simulink model "Lab2_simulink_plc"  (R2023b)
settings: Solver=FixedStepAuto  StartTime=0.0  StopTime=inf  RelTol=1e-3
InitFcn:
  | d13_init.PRE = 50;
undefined symbols (not defined in the file or in its folder): BOOL, DINT, OTL, slplc
libraries: arduinolib x9
(part 3 of 31)

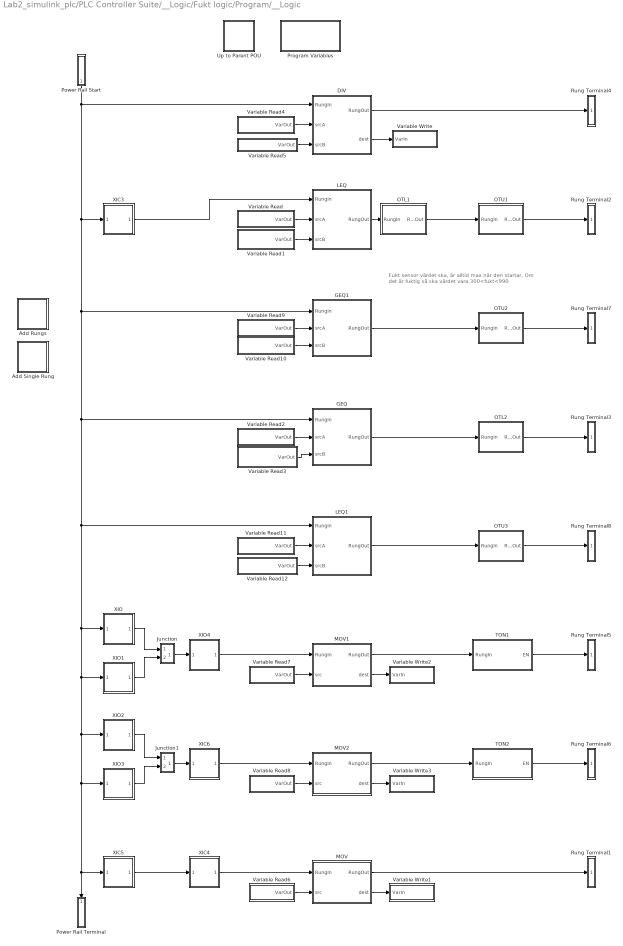
== system Lab2_simulink_plc/PLC Controller Suite/__Logic/Fukt logic/Program/__Logic ==
  note: Fukt sensor värdet ska, är alltid max när den startar,
      | Om det är fuktig så ska värdet vara 300<fukt<990
  Add Rungs : Subsystem  DisableCoverage=on  TreatAsAtomicUnit=on  mask{PLCRungNumberToAdd=2, PLCBlockType=AddRungs}
      mask prompts: PLCRungNumberToAdd "Rung Number:"
      mask Help: web([docroot '/plccoder/ref/addrungs' '.html']);
      mask DialogControl PLCAddRungs Prompt: Add Rungs
      mask DialogControl PLCRungAddButton Prompt: Add
      mask DialogControl PLCRungAddButton Callback: slplc.callbacks.addLadderRungs(gcb);
      mask Display: disp(sprintf(['Add' newline 'Rungs']));
  Add Single Rung : Subsystem  DisableCoverage=on  TreatAsAtomicUnit=on  mask{PLCRungNumberToAdd=1, PLCBlockType=AddSingleRung}
      settings: OpenFcn=slplc.callbacks.addLadderRungs(gcb);
      mask Help: web([docroot '/plccoder/ref/addsinglerung' '.html']);
      mask DialogControl PLCAddRung Prompt: Add Single Rung
      mask Display: disp(sprintf(['Add' newline '1 Rung']));
  DIV : Subsystem  Priority=4  TreatAsAtomicUnit=on  mask{PLCBlockType=DIV, PLCPOUType=stdFC}
      mask Help: web([docroot '/plccoder/ref/div' '.html']);
      mask DialogControl PLCBlockTitle Prompt: Divide (DIV)
      mask DialogControl PLCBlockDesc Prompt: Divide Source A by Source B.
      mask Display: disp('DIV');
  GEQ : Subsystem  Priority=21  TreatAsAtomicUnit=on  mask{PLCBlockType=GEQ, PLCPOUType=stdFC}
      mask Help: web([docroot '/plccoder/ref/geq' '.html']);
      mask DialogControl PLCBlockTitle Prompt: Greater Than or Equal To (GEQ)
      mask DialogControl PLCBlockDesc Prompt: Test whether Source A is greater than or equal to Source B.
      mask Display: disp('GEQ');
  GEQ1 : Subsystem  Priority=16  TreatAsAtomicUnit=on  mask{PLCBlockType=GEQ, PLCPOUType=stdFC}
      mask Help: web([docroot '/plccoder/ref/geq' '.html']);
      mask DialogControl PLCBlockTitle Prompt: Greater Than or Equal To (GEQ)
      mask DialogControl PLCBlockDesc Prompt: Test whether Source A is greater than or equal to Source B.
      mask Display: disp('GEQ');
  Junction : Subsystem  Priority=31  DisableCoverage=on  TreatAsAtomicUnit=on  mask{PLCBlockType=Junction, PLCPOUType=BranchJunction}
      mask Help: web([docroot '/plccoder/ref/junction' '.html']);
      mask DialogControl PLCBlockTitle Prompt: Rung Merge Point
      mask DialogControl PLCBlockDesc Prompt: This is the ladder diagram rung merge point.
  Junction1 : Subsystem  Priority=40  DisableCoverage=on  TreatAsAtomicUnit=on  mask{PLCBlockType=Junction, PLCPOUType=BranchJunction}
      mask Help: web([docroot '/plccoder/ref/junction' '.html']);
      mask DialogControl PLCBlockTitle Prompt: Rung Merge Point
      mask DialogControl PLCBlockDesc Prompt: This is the ladder diagram rung merge point.
  LEQ : Subsystem  Priority=10  TreatAsAtomicUnit=on  mask{PLCBlockType=LEQ, PLCPOUType=stdFC}
      mask Help: web([docroot '/plccoder/ref/leq' '.html']);
      mask DialogControl PLCBlockTitle Prompt: Less Than or Equal To (LEQ)
      mask DialogControl PLCBlockDesc Prompt: Test whether Source A is less than or equal to Source B.
      mask Display: disp('LEQ');
  LEQ1 : Subsystem  Priority=26  TreatAsAtomicUnit=on  mask{PLCBlockType=LEQ, PLCPOUType=stdFC}
      mask Help: web([docroot '/plccoder/ref/leq' '.html']);
      mask DialogControl PLCBlockTitle Prompt: Less Than or Equal To (LEQ)
      mask DialogControl PLCBlockDesc Prompt: Test whether Source A is less than or equal to Source B.
      mask Display: disp('LEQ');
  MOV : Subsystem  Priority=50  TreatAsAtomicUnit=on  mask{PLCBlockType=MOV, PLCPOUType=stdFC}
      mask Help: web([docroot '/plccoder/ref/mov' '.html']);
      mask DialogControl PLCBlockTitle Prompt: Move (MOV)
      mask DialogControl PLCBlockDesc Prompt: Move a copy of the Source to the Destination. The Source remains unchanged.
      mask Display: disp('MOV');
  MOV1 : Subsystem  Priority=34  TreatAsAtomicUnit=on  mask{PLCBlockType=MOV, PLCPOUType=stdFC}
      mask Help: web([docroot '/plccoder/ref/mov' '.html']);
      mask DialogControl PLCBlockTitle Prompt: Move (MOV)
      mask DialogControl PLCBlockDesc Prompt: Move a copy of the Source to the Destination. The Source remains unchanged.
      mask Display: disp('MOV');
  MOV2 : Subsystem  Priority=43  TreatAsAtomicUnit=on  mask{PLCBlockType=MOV, PLCPOUType=stdFC}
      mask Help: web([docroot '/plccoder/ref/mov' '.html']);
      mask DialogControl PLCBlockTitle Prompt: Move (MOV)
      mask DialogControl PLCBlockDesc Prompt: Move a copy of the Source to the Destination. The Source remains unchanged.
      mask Display: disp('MOV');
  OTL1 : Subsystem  Priority=11  TreatAsAtomicUnit=on  mask{PLCOperandTag=fukt_low, PLCBlockType=OTL, PLCPOUType=Coil, PLCOperandExpression=xxx_PLC_VAR_fukt_low}
      mask prompts: PLCOperandTag "Operand Tag:"
      mask init:
      | slplc.callbacks.initInstruction(gcb);
      settings: AttributesFormatString=%<PLCOperandTag>
      mask Help: web([docroot '/plccoder/ref/otl' '.html']);
      mask DialogControl PLCBlockTitle Prompt: Output Latch (OTL)
      mask Display: disp('L');
  OTL2 : Subsystem  Priority=22  TreatAsAtomicUnit=on  mask{PLCOperandTag=fukt_high, PLCBlockType=OTL, PLCPOUType=Coil, PLCOperandExpression=xxx_PLC_VAR_fukt_high}
      mask prompts: PLCOperandTag "Operand Tag:"
      mask init:
      | slplc.callbacks.initInstruction(gcb);
      settings: AttributesFormatString=%<PLCOperandTag>
      mask Help: web([docroot '/plccoder/ref/otl' '.html']);
      mask DialogControl PLCBlockTitle Prompt: Output Latch (OTL)
      mask Display: disp('L');
  OTU1 : Subsystem  Priority=12  TreatAsAtomicUnit=on  mask{PLCOperandTag=fukt_polling, PLCBlockType=OTU, PLCPOUType=Coil, PLCOperandExpression=xxx_PLC_VAR_fukt_polling}
      mask prompts: PLCOperandTag "Operand Tag:"
      mask init:
      | slplc.callbacks.initInstruction(gcb);
      settings: AttributesFormatString=%<PLCOperandTag>
      mask Help: web([docroot '/plccoder/ref/otu' '.html']);
      mask DialogControl PLCBlockTitle Prompt: Output Unlatch (OTU)
      mask Display: disp('U');
  OTU2 : Subsystem  Priority=17  TreatAsAtomicUnit=on  mask{PLCOperandTag=fukt_low, PLCBlockType=OTU, PLCPOUType=Coil, PLCOperandExpression=xxx_PLC_VAR_fukt_low}
      mask prompts: PLCOperandTag "Operand Tag:"
      mask init:
      | slplc.callbacks.initInstruction(gcb);
      settings: AttributesFormatString=%<PLCOperandTag>
      mask Help: web([docroot '/plccoder/ref/otu' '.html']);
      mask DialogControl PLCBlockTitle Prompt: Output Unlatch (OTU)
      mask Display: disp('U');
  OTU3 : Subsystem  Priority=27  TreatAsAtomicUnit=on  mask{PLCOperandTag=fukt_high, PLCBlockType=OTU, PLCPOUType=Coil, PLCOperandExpression=xxx_PLC_VAR_fukt_high}
      mask prompts: PLCOperandTag "Operand Tag:"
      mask init:
      | slplc.callbacks.initInstruction(gcb);
      settings: AttributesFormatString=%<PLCOperandTag>
      mask Help: web([docroot '/plccoder/ref/otu' '.html']);
      mask DialogControl PLCBlockTitle Prompt: Output Unlatch (OTU)
      mask Display: disp('U');
  Power Rail Start : Subsystem  Priority=1  DisableCoverage=on  TreatAsAtomicUnit=on  mask{PLCBlockType=PowerRailStart}
      mask Help: web([docroot '/plccoder/ref/powerrailstart' '.html']);
      mask DialogControl PLCBlockTitle Prompt: Power Rail Start
      mask DialogControl PLCBlockDesc Prompt: This is the ladder diagram left power rail start block.
  Power Rail Terminal : Subsystem  DisableCoverage=on  TreatAsAtomicUnit=on  mask{PLCBlockType=PowerRailTerminal}
      mask Help: web([docroot '/plccoder/ref/powerrailterminal' '.html']);
      mask DialogControl PLCBlockTitle Prompt: Power Rail Terminal
      mask DialogControl PLCBlockDesc Prompt: This is the ladder diagram left power rail terminal block.
  Program Variables : Subsystem  Tag=ProgramVariableSS  DisableCoverage=on  TreatAsAtomicUnit=on  mask{PLCVarFukt_constant_highName=Fukt_constant_high, PLCVarFukt_constant_highScope=Local, PLCVarFukt_constant_highPortType=Hidden, PLCVarFukt_constant_highPortIndex=12, PLCVarFukt_constant_highDataType=DINT, PLCVarFukt_constant_highSize=1, PLCVarFukt_constant_highInitialValue=900, PLCVarFukt_constant_highToDelete=off, PLCVarFukt_constant_lowName=Fukt_constant_low, PLCVarFukt_constant_lowScope=Local, PLCVarFukt_constant_lowPortType=Hidden, PLCVarFukt_constant_lowPortIndex=12, PLCVarFukt_constant_lowDataType=DINT, PLCVarFukt_constant_lowSize=1, PLCVarFukt_constant_lowInitialValue=300, PLCVarFukt_constant_lowToDelete=off, PLCVarconstantName=constant, PLCVarconstantScope=Local, PLCVarconstantPortType=Hidden, PLCVarconstantPortIndex=12, PLCVarconstantDataType=uint16, PLCVarconstantSize=1, PLCVarconstantInitialValue=4, PLCVarconstantToDelete=off, PLCVarduty_cycleName=duty_cycle, PLCVarduty_cycleScope=Local, PLCVarduty_cyclePortType=Hidden, PLCVarduty_cyclePortIndex=12, PLCVarduty_cycleDataType=uint8, PLCVarduty_cycleSize=1, PLCVarduty_cycleInitialValue=0, PLCVarduty_cycleToDelete=off, PLCVarfukt_highName=fukt_high, PLCVarfukt_highScope=Local, PLCVarfukt_highPortType=Hidden, PLCVarfukt_highPortIndex=12, PLCVarfukt_highDataType=BOOL, PLCVarfukt_highSize=1, PLCVarfukt_highInitialValue=false, PLCVarfukt_highToDelete=off, PLCVarfukt_lowName=fukt_low, PLCVarfukt_lowScope=Local, PLCVarfukt_lowPortType=Hidden, PLCVarfukt_lowPortIndex=12, PLCVarfukt_lowDataType=BOOL, PLCVarfukt_lowSize=1, PLCVarfukt_lowInitialValue=false, PLCVarfukt_lowToDelete=off, PLCVarorange_led_timer_offName=orange_led_timer_off, PLCVarorange_led_timer_offScope=Local, PLCVarorange_led_timer_offPortType=Hidden, PLCVarorange_led_timer_offPortIndex=12, PLCVarorange_led_timer_offDataType=Bus: TIMER, PLCVarorange_led_timer_offSize=1, PLCVarorange_led_timer_offInitialValue=orange_period, PLCVarorange_led_timer_offToDelete=off, PLCVarorange_led_timer_onName=orange_led_timer_on, PLCVarorange_led_timer_onScope=Local, PLCVarorange_led_timer_onPortType=Hidden, PLCVarorange_led_timer_onPortIndex=12, PLCVarorange_led_timer_onDataType=Bus: TIMER, PLCVarorange_led_timer_onSize=1, PLCVarorange_led_timer_onInitialValue=orange_period, PLCVarorange_led_timer_onToDelete=off, PLCVarorange_offName=orange_off, PLCVarorange_offScope=Local, PLCVarorange_offPortType=Hidden, PLCVarorange_offPortIndex=12, PLCVarorange_offDataType=uint8, PLCVarorange_offSize=1, PLCVarorange_offInitialValue=0, PLCVarorange_offToDelete=off, PLCVarFukt_value_copyName=Fukt_value_copy, PLCVarFukt_value_copyScope=External, PLCVarFukt_value_copyPortType=Hidden, PLCVarFukt_value_copyPortIndex=12, PLCVarFukt_value_copyDataType=DINT, PLCVarFukt_value_copySize=-1, PLCVarFukt_value_copyInitialValue=0, PLCVarFukt_value_copyToDelete=off, PLCVarOrangeLedName=OrangeLed, PLCVarOrangeLedScope=External, PLCVarOrangeLedPortType=Hidden, PLCVarOrangeLedPortIndex=12, PLCVarOrangeLedDataType=DINT, PLCVarOrangeLedSize=-1, PLCVarOrangeLedInitialValue=0, PLCVarOrangeLedToDelete=off, PLCVarfukt_pollingName=fukt_polling, PLCVarfukt_pollingScope=External, PLCVarfukt_pollingPortType=Hidden, PLCVarfukt_pollingPortIndex=12, PLCVarfukt_pollingDataType=DINT, PLCVarfukt_pollingSize=-1, PLCVarfukt_pollingInitialValue=0, PLCVarfukt_pollingToDelete=off}
      mask init:
      | slplc.callbacks.initPOUVarSS(gcb);
      settings: OpenFcn=slplc.callbacks.openProgramVariableSS(gcb);
      mask Help: web([docroot '/plccoder/ref/programvariables' '.html']);
      mask TypeOptions PLCVarFukt_constant_highScope: Local | External
      mask Callback PLCVarFukt_constant_highScope: slplc.callbacks.updatePOUVarSS(gcb);
      mask TypeOptions PLCVarFukt_constant_highPortType: Hidden | Inport | Outport
      mask Callback PLCVarFukt_constant_highPortType: slplc.callbacks.updatePOUVarSS(gcb);
      mask TypeOptions PLCVarFukt_constant_highPortIndex: 1 | 2 | 3 | 4 | 5 | 6 | 7 | 8 | 9 | 10 | 11 | 12
      mask TypeOptions PLCVarFukt_constant_lowScope: Local | External
      mask Callback PLCVarFukt_constant_lowScope: slplc.callbacks.updatePOUVarSS(gcb);
      mask TypeOptions PLCVarFukt_constant_lowPortType: Hidden | Inport | Outport
      mask Callback PLCVarFukt_constant_lowPortType: slplc.callbacks.updatePOUVarSS(gcb);
      mask TypeOptions PLCVarFukt_constant_lowPortIndex: 1 | 2 | 3 | 4 | 5 | 6 | 7 | 8 | 9 | 10 | 11 | 12
      mask TypeOptions PLCVarconstantScope: Local | External
      mask Callback PLCVarconstantScope: slplc.callbacks.updatePOUVarSS(gcb);
      mask TypeOptions PLCVarconstantPortType: Hidden | Inport | Outport
      mask Callback PLCVarconstantPortType: slplc.callbacks.updatePOUVarSS(gcb);
      mask TypeOptions PLCVarconstantPortIndex: 1 | 2 | 3 | 4 | 5 | 6 | 7 | 8 | 9 | 10 | 11 | 12
      mask TypeOptions PLCVarduty_cycleScope: Local | External
      mask Callback PLCVarduty_cycleScope: slplc.callbacks.updatePOUVarSS(gcb);
      mask TypeOptions PLCVarduty_cyclePortType: Hidden | Inport | Outport
      mask Callback PLCVarduty_cyclePortType: slplc.callbacks.updatePOUVarSS(gcb);
      mask TypeOptions PLCVarduty_cyclePortIndex: 1 | 2 | 3 | 4 | 5 | 6 | 7 | 8 | 9 | 10 | 11 | 12
      mask TypeOptions PLCVarfukt_highScope: Local | External
      mask Callback PLCVarfukt_highScope: slplc.callbacks.updatePOUVarSS(gcb);
      mask TypeOptions PLCVarfukt_highPortType: Hidden | Inport | Outport
      mask Callback PLCVarfukt_highPortType: slplc.callbacks.updatePOUVarSS(gcb);
      mask TypeOptions PLCVarfukt_highPortIndex: 1 | 2 | 3 | 4 | 5 | 6 | 7 | 8 | 9 | 10 | 11 | 12
      mask TypeOptions PLCVarfukt_lowScope: Local | External
      mask Callback PLCVarfukt_lowScope: slplc.callbacks.updatePOUVarSS(gcb);
      mask TypeOptions PLCVarfukt_lowPortType: Hidden | Inport | Outport
      mask Callback PLCVarfukt_lowPortType: slplc.callbacks.updatePOUVarSS(gcb);
      mask TypeOptions PLCVarfukt_lowPortIndex: 1 | 2 | 3 | 4 | 5 | 6 | 7 | 8 | 9 | 10 | 11 | 12
      mask TypeOptions PLCVarorange_led_timer_offScope: Local | External
      mask Callback PLCVarorange_led_timer_offScope: slplc.callbacks.updatePOUVarSS(gcb);
      mask TypeOptions PLCVarorange_led_timer_offPortType: Hidden | Inport | Outport
      mask Callback PLCVarorange_led_timer_offPortType: slplc.callbacks.updatePOUVarSS(gcb);
      mask TypeOptions PLCVarorange_led_timer_offPortIndex: 1 | 2 | 3 | 4 | 5 | 6 | 7 | 8 | 9 | 10 | 11 | 12
      mask TypeOptions PLCVarorange_led_timer_onScope: Local | External
      mask Callback PLCVarorange_led_timer_onScope: slplc.callbacks.updatePOUVarSS(gcb);
      mask TypeOptions PLCVarorange_led_timer_onPortType: Hidden | Inport | Outport
      mask Callback PLCVarorange_led_timer_onPortType: slplc.callbacks.updatePOUVarSS(gcb);
      mask TypeOptions PLCVarorange_led_timer_onPortIndex: 1 | 2 | 3 | 4 | 5 | 6 | 7 | 8 | 9 | 10 | 11 | 12
      mask TypeOptions PLCVarorange_offScope: Local | External
      mask Callback PLCVarorange_offScope: slplc.callbacks.updatePOUVarSS(gcb);
      mask TypeOptions PLCVarorange_offPortType: Hidden | Inport | Outport
      mask Callback PLCVarorange_offPortType: slplc.callbacks.updatePOUVarSS(gcb);
      mask TypeOptions PLCVarorange_offPortIndex: 1 | 2 | 3 | 4 | 5 | 6 | 7 | 8 | 9 | 10 | 11 | 12
      mask TypeOptions PLCVarFukt_value_copyScope: Local | External
      mask Callback PLCVarFukt_value_copyScope: slplc.callbacks.updatePOUVarSS(gcb);
      mask TypeOptions PLCVarFukt_value_copyPortType: Hidden | Inport | Outport
      mask Callback PLCVarFukt_value_copyPortType: slplc.callbacks.updatePOUVarSS(gcb);
      mask TypeOptions PLCVarFukt_value_copyPortIndex: 1 | 2 | 3 | 4 | 5 | 6 | 7 | 8 | 9 | 10 | 11 | 12
      mask TypeOptions PLCVarOrangeLedScope: Local | External
      mask Callback PLCVarOrangeLedScope: slplc.callbacks.updatePOUVarSS(gcb);
      mask TypeOptions PLCVarOrangeLedPortType: Hidden | Inport | Outport
      mask Callback PLCVarOrangeLedPortType: slplc.callbacks.updatePOUVarSS(gcb);
      mask TypeOptions PLCVarOrangeLedPortIndex: 1 | 2 | 3 | 4 | 5 | 6 | 7 | 8 | 9 | 10 | 11 | 12
      mask TypeOptions PLCVarfukt_pollingScope: Local | External
      mask Callback PLCVarfukt_pollingScope: slplc.callbacks.updatePOUVarSS(gcb);
      mask TypeOptions PLCVarfukt_pollingPortType: Hidden | Inport | Outport
      mask Callback PLCVarfukt_pollingPortType: slplc.callbacks.updatePOUVarSS(gcb);
      mask TypeOptions PLCVarfukt_pollingPortIndex: 1 | 2 | 3 | 4 | 5 | 6 | 7 | 8 | 9 | 10 | 11 | 12
      mask DialogControl PLCBlockTitle Prompt: Program Variable Spreadsheet
      mask DialogControl PLCVarListHeaderName Prompt: Name
      mask DialogControl PLCVarListHeaderScope Prompt: Scope
      mask DialogControl PLCVarListHeaderDataType Prompt: Data Type
      mask DialogControl PLCVarListHeaderSize Prompt: Size
      mask DialogControl PLCVarListHeaderInitialValue Prompt: Initial Value
      mask DialogControl PLCVarListHeaderToDelete Prompt: Delete
  Rung Terminal1 : Subsystem  Priority=52  DisableCoverage=on  TreatAsAtomicUnit=on  mask{PLCRungTerminalIndex=8, PLCBlockType=RungTerminal}
      settings: AttributesFormatString=Rung %<PLCRungTerminalIndex>
      mask Help: web([docroot '/plccoder/ref/rungterminal' '.html']);
      mask DialogControl PLCBlockTitle Prompt: Rung Terminal
      mask DialogControl PLCBlockDescText Prompt: This is the ladder diagram rung terminal.
  Rung Terminal2 : Subsystem  Priority=13  DisableCoverage=on  TreatAsAtomicUnit=on  mask{PLCRungTerminalIndex=2, PLCBlockType=RungTerminal}
      settings: AttributesFormatString=Rung %<PLCRungTerminalIndex>
      mask Help: web([docroot '/plccoder/ref/rungterminal' '.html']);
      mask DialogControl PLCBlockTitle Prompt: Rung Terminal
      mask DialogControl PLCBlockDescText Prompt: This is the ladder diagram rung terminal.
  Rung Terminal3 : Subsystem  Priority=23  DisableCoverage=on  TreatAsAtomicUnit=on  mask{PLCRungTerminalIndex=4, PLCBlockType=RungTerminal}
      settings: AttributesFormatString=Rung %<PLCRungTerminalIndex>
      mask Help: web([docroot '/plccoder/ref/rungterminal' '.html']);
      mask DialogControl PLCBlockTitle Prompt: Rung Terminal
      mask DialogControl PLCBlockDescText Prompt: This is the ladder diagram rung terminal.
  Rung Terminal4 : Subsystem  Priority=6  DisableCoverage=on  TreatAsAtomicUnit=on  mask{PLCRungTerminalIndex=1, PLCBlockType=RungTerminal}
      settings: AttributesFormatString=Rung %<PLCRungTerminalIndex>
      mask Help: web([docroot '/plccoder/ref/rungterminal' '.html']);
      mask DialogControl PLCBlockTitle Prompt: Rung Terminal
      mask DialogControl PLCBlockDescText Prompt: This is the ladder diagram rung terminal.
  Rung Terminal5 : Subsystem  Priority=37  DisableCoverage=on  TreatAsAtomicUnit=on  mask{PLCRungTerminalIndex=6, PLCBlockType=RungTerminal}
      settings: AttributesFormatString=Rung %<PLCRungTerminalIndex>
      mask Help: web([docroot '/plccoder/ref/rungterminal' '.html']);
      mask DialogControl PLCBlockTitle Prompt: Rung Terminal
      mask DialogControl PLCBlockDescText Prompt: This is the ladder diagram rung terminal.
  Rung Terminal6 : Subsystem  Priority=46  DisableCoverage=on  TreatAsAtomicUnit=on  mask{PLCRungTerminalIndex=7, PLCBlockType=RungTerminal}
      settings: AttributesFormatString=Rung %<PLCRungTerminalIndex>
      mask Help: web([docroot '/plccoder/ref/rungterminal' '.html']);
      mask DialogControl PLCBlockTitle Prompt: Rung Terminal
      mask DialogControl PLCBlockDescText Prompt: This is the ladder diagram rung terminal.
  Rung Terminal7 : Subsystem  Priority=18  DisableCoverage=on  TreatAsAtomicUnit=on  mask{PLCRungTerminalIndex=3, PLCBlockType=RungTerminal}
      settings: AttributesFormatString=Rung %<PLCRungTerminalIndex>
      mask Help: web([docroot '/plccoder/ref/rungterminal' '.html']);
      mask DialogControl PLCBlockTitle Prompt: Rung Terminal
      mask DialogControl PLCBlockDescText Prompt: This is the ladder diagram rung terminal.
  Rung Terminal8 : Subsystem  Priority=28  DisableCoverage=on  TreatAsAtomicUnit=on  mask{PLCRungTerminalIndex=5, PLCBlockType=RungTerminal}
      settings: AttributesFormatString=Rung %<PLCRungTerminalIndex>
      mask Help: web([docroot '/plccoder/ref/rungterminal' '.html']);
      mask DialogControl PLCBlockTitle Prompt: Rung Terminal
      mask DialogControl PLCBlockDescText Prompt: This is the ladder diagram rung terminal.
  TON1 : Subsystem  Priority=36  TreatAsAtomicUnit=on  mask{PLCOperandTag=orange_led_timer_on, PLCTimerSampleTime=0.2, PLCBlockType=TON, PLCPOUType=stdFB, PLCOperandAccess=ReadWrite, PLCOperandExpression=xxx_PLC_VAR_orange_led_timer_on}
      mask prompts: PLCOperandTag "Timer Tag:"; PLCTimerSampleTime "Timer Sample Time:"
      mask init:
      | block = gcb;
      | maskObj = Simulink.Mask.get(block);
      | maskObj.Display = sprintf('disp([''%s'' newline newline ''%s'']);', PLCBlockType, PLCOperandTag);
      | slplc.callbacks.initInstruction(block);
      settings: AttributesFormatString=%<PLCBlockType>: %<PLCOperandTag>
      mask Help: web([docroot '/plccoder/ref/ton' '.html']);
      mask DialogControl PLCBlockTitle Prompt: Timer On Delay (TON)
      mask Display: disp(['TON' newline newline 'orange_led_timer_on']);
  TON2 : Subsystem  Priority=45  TreatAsAtomicUnit=on  mask{PLCOperandTag=orange_led_timer_off, PLCTimerSampleTime=0.2, PLCBlockType=TON, PLCPOUType=stdFB, PLCOperandAccess=ReadWrite, PLCOperandExpression=xxx_PLC_VAR_orange_led_timer_off}
      mask prompts: PLCOperandTag "Timer Tag:"; PLCTimerSampleTime "Timer Sample Time:"
      mask init:
      | block = gcb;
      | maskObj = Simulink.Mask.get(block);
      | maskObj.Display = sprintf('disp([''%s'' newline newline ''%s'']);', PLCBlockType, PLCOperandTag);
      | slplc.callbacks.initInstruction(block);
      settings: AttributesFormatString=%<PLCBlockType>: %<PLCOperandTag>
      mask Help: web([docroot '/plccoder/ref/ton' '.html']);
      mask DialogControl PLCBlockTitle Prompt: Timer On Delay (TON)
      mask Display: disp(['TON' newline newline 'orange_led_timer_off']);
  Up to Parent POU : Subsystem  DisableCoverage=on  TreatAsAtomicUnit=on  mask{PLCBlockType=OpenParentPOU}
      settings: OpenFcn=slplc.callbacks.openParentPOUBlock(gcb);
      mask Help: web([docroot '/plccoder/ref/uptoparentpou' '.html']);
      mask DialogControl PLCBlockTitle Prompt: Add Single Rung
  Variable Read : Subsystem  Priority=8  TreatAsAtomicUnit=on  mask{PLCOperandTag=Fukt_value_copy, PLCBlockType=VariableRead, PLCOperandAccess=Read, PLCPOUType=VariableRead, PLCOperandExpression=xxx_PLC_VAR_Fukt_value_copy}
      mask prompts: PLCOperandTag "Data Tag:"
      mask init:
      | block = gcb;
      | maskObj = Simulink.Mask.get(block);
      | maskObj.Display = sprintf('disp(''%s'');', PLCOperandTag);
      | slplc.callbacks.initInstruction(block);
      mask Help: web([docroot '/plccoder/ref/variableread' '.html']);
      mask DialogControl PLCBlockTitle Prompt: Data Tag Read
      mask Display: disp('Fukt_value_copy');
  Variable Read1 : Subsystem  Priority=9  TreatAsAtomicUnit=on  mask{PLCOperandTag=Fukt_constant_high, PLCBlockType=VariableRead, PLCOperandAccess=Read, PLCPOUType=VariableRead, PLCOperandExpression=xxx_PLC_VAR_Fukt_constant_high}
      mask prompts: PLCOperandTag "Data Tag:"
      mask init:
      | block = gcb;
      | maskObj = Simulink.Mask.get(block);
      | maskObj.Display = sprintf('disp(''%s'');', PLCOperandTag);
      | slplc.callbacks.initInstruction(block);
      mask Help: web([docroot '/plccoder/ref/variableread' '.html']);
      mask DialogControl PLCBlockTitle Prompt: Data Tag Read
      mask Display: disp('Fukt_constant_high');
  Variable Read10 : Subsystem  Priority=15  TreatAsAtomicUnit=on  mask{PLCOperandTag=Fukt_constant_high, PLCBlockType=VariableRead, PLCOperandAccess=Read, PLCPOUType=VariableRead, PLCOperandExpression=xxx_PLC_VAR_Fukt_constant_high}
      mask prompts: PLCOperandTag "Data Tag:"
      mask init:
      | block = gcb;
      | maskObj = Simulink.Mask.get(block);
      | maskObj.Display = sprintf('disp(''%s'');', PLCOperandTag);
      | slplc.callbacks.initInstruction(block);
      mask Help: web([docroot '/plccoder/ref/variableread' '.html']);
      mask DialogControl PLCBlockTitle Prompt: Data Tag Read
      mask Display: disp('Fukt_constant_high');
  Variable Read11 : Subsystem  Priority=24  TreatAsAtomicUnit=on  mask{PLCOperandTag=Fukt_value_copy, PLCBlockType=VariableRead, PLCOperandAccess=Read, PLCPOUType=VariableRead, PLCOperandExpression=xxx_PLC_VAR_Fukt_value_copy}
      mask prompts: PLCOperandTag "Data Tag:"
      mask init:
      | block = gcb;
      | maskObj = Simulink.Mask.get(block);
      | maskObj.Display = sprintf('disp(''%s'');', PLCOperandTag);
      | slplc.callbacks.initInstruction(block);
      mask Help: web([docroot '/plccoder/ref/variableread' '.html']);
      mask DialogControl PLCBlockTitle Prompt: Data Tag Read
      mask Display: disp('Fukt_value_copy');
  Variable Read12 : Subsystem  Priority=25  TreatAsAtomicUnit=on  mask{PLCOperandTag=Fukt_constant_low, PLCBlockType=VariableRead, PLCOperandAccess=Read, PLCPOUType=VariableRead, PLCOperandExpression=xxx_PLC_VAR_Fukt_constant_low}
      mask prompts: PLCOperandTag "Data Tag:"
      mask init:
      | block = gcb;
      | maskObj = Simulink.Mask.get(block);
      | maskObj.Display = sprintf('disp(''%s'');', PLCOperandTag);
      | slplc.callbacks.initInstruction(block);
      mask Help: web([docroot '/plccoder/ref/variableread' '.html']);
      mask DialogControl PLCBlockTitle Prompt: Data Tag Read
      mask Display: disp('Fukt_constant_low');
  Variable Read2 : Subsystem  Priority=19  TreatAsAtomicUnit=on  mask{PLCOperandTag=Fukt_value_copy, PLCBlockType=VariableRead, PLCOperandAccess=Read, PLCPOUType=VariableRead, PLCOperandExpression=xxx_PLC_VAR_Fukt_value_copy}
      mask prompts: PLCOperandTag "Data Tag:"
      mask init:
      | block = gcb;
      | maskObj = Simulink.Mask.get(block);
      | maskObj.Display = sprintf('disp(''%s'');', PLCOperandTag);
      | slplc.callbacks.initInstruction(block);
      mask Help: web([docroot '/plccoder/ref/variableread' '.html']);
      mask DialogControl PLCBlockTitle Prompt: Data Tag Read
      mask Display: disp('Fukt_value_copy');
  Variable Read3 : Subsystem  Priority=20  TreatAsAtomicUnit=on  mask{PLCOperandTag=Fukt_constant_low, PLCBlockType=VariableRead, PLCOperandAccess=Read, PLCPOUType=VariableRead, PLCOperandExpression=xxx_PLC_VAR_Fukt_constant_low}
      mask prompts: PLCOperandTag "Data Tag:"
      mask init:
      | block = gcb;
      | maskObj = Simulink.Mask.get(block);
      | maskObj.Display = sprintf('disp(''%s'');', PLCOperandTag);
      | slplc.callbacks.initInstruction(block);
      mask Help: web([docroot '/plccoder/ref/variableread' '.html']);
      mask DialogControl PLCBlockTitle Prompt: Data Tag Read
      mask Display: disp('Fukt_constant_low');
  Variable Read4 : Subsystem  Priority=2  TreatAsAtomicUnit=on  mask{PLCOperandTag=Fukt_value_copy, PLCBlockType=VariableRead, PLCOperandAccess=Read, PLCPOUType=VariableRead, PLCOperandExpression=xxx_PLC_VAR_Fukt_value_copy}
      mask prompts: PLCOperandTag "Data Tag:"
      mask init:
      | block = gcb;
      | maskObj = Simulink.Mask.get(block);
      | maskObj.Display = sprintf('disp(''%s'');', PLCOperandTag);
      | slplc.callbacks.initInstruction(block);
      mask Help: web([docroot '/plccoder/ref/variableread' '.html']);
      mask DialogControl PLCBlockTitle Prompt: Data Tag Read
      mask Display: disp('Fukt_value_copy');
  Variable Read5 : Subsystem  Priority=3  TreatAsAtomicUnit=on  mask{PLCOperandTag=constant, PLCBlockType=VariableRead, PLCOperandAccess=Read, PLCPOUType=VariableRead, PLCOperandExpression=xxx_PLC_VAR_constant}
      mask prompts: PLCOperandTag "Data Tag:"
      mask init:
      | block = gcb;
      | maskObj = Simulink.Mask.get(block);
      | maskObj.Display = sprintf('disp(''%s'');', PLCOperandTag);
      | slplc.callbacks.initInstruction(block);
      mask Help: web([docroot '/plccoder/ref/variableread' '.html']);
      mask DialogControl PLCBlockTitle Prompt: Data Tag Read
      mask Display: disp('constant');
  Variable Read6 : Subsystem  Priority=49  TreatAsAtomicUnit=on  mask{PLCOperandTag=duty_cycle, PLCBlockType=VariableRead, PLCOperandAccess=Read, PLCPOUType=VariableRead, PLCOperandExpression=xxx_PLC_VAR_duty_cycle}
      mask prompts: PLCOperandTag "Data Tag:"
      mask init:
      | block = gcb;
      | maskObj = Simulink.Mask.get(block);
      | maskObj.Display = sprintf('disp(''%s'');', PLCOperandTag);
      | slplc.callbacks.initInstruction(block);
      mask Help: web([docroot '/plccoder/ref/variableread' '.html']);
      mask DialogControl PLCBlockTitle Prompt: Data Tag Read
      mask Display: disp('duty_cycle');
  Variable Read7 : Subsystem  Priority=33  TreatAsAtomicUnit=on  mask{PLCOperandTag=duty_cycle, PLCBlockType=VariableRead, PLCOperandAccess=Read, PLCPOUType=VariableRead, PLCOperandExpression=xxx_PLC_VAR_duty_cycle}
      mask prompts: PLCOperandTag "Data Tag:"
      mask init:
      | block = gcb;
      | maskObj = Simulink.Mask.get(block);
      | maskObj.Display = sprintf('disp(''%s'');', PLCOperandTag);
      | slplc.callbacks.initInstruction(block);
      mask Help: web([docroot '/plccoder/ref/variableread' '.html']);
      mask DialogControl PLCBlockTitle Prompt: Data Tag Read
      mask Display: disp('duty_cycle');
  Variable Read8 : Subsystem  Priority=42  TreatAsAtomicUnit=on  mask{PLCOperandTag=orange_off, PLCBlockType=VariableRead, PLCOperandAccess=Read, PLCPOUType=VariableRead, PLCOperandExpression=xxx_PLC_VAR_orange_off}
      mask prompts: PLCOperandTag "Data Tag:"
      mask init:
      | block = gcb;
      | maskObj = Simulink.Mask.get(block);
      | maskObj.Display = sprintf('disp(''%s'');', PLCOperandTag);
      | slplc.callbacks.initInstruction(block);
      mask Help: web([docroot '/plccoder/ref/variableread' '.html']);
      mask DialogControl PLCBlockTitle Prompt: Data Tag Read
      mask Display: disp('orange_off');
  Variable Read9 : Subsystem  Priority=14  TreatAsAtomicUnit=on  mask{PLCOperandTag=Fukt_value_copy, PLCBlockType=VariableRead, PLCOperandAccess=Read, PLCPOUType=VariableRead, PLCOperandExpression=xxx_PLC_VAR_Fukt_value_copy}
      mask prompts: PLCOperandTag "Data Tag:"
      mask init:
      | block = gcb;
      | maskObj = Simulink.Mask.get(block);
      | maskObj.Display = sprintf('disp(''%s'');', PLCOperandTag);
      | slplc.callbacks.initInstruction(block);
      mask Help: web([docroot '/plccoder/ref/variableread' '.html']);
      mask DialogControl PLCBlockTitle Prompt: Data Tag Read
      mask Display: disp('Fukt_value_copy');
  Variable Write : Subsystem  Priority=5  TreatAsAtomicUnit=on  mask{PLCOperandTag=duty_cycle, PLCBlockType=VariableWrite, PLCOperandAccess=Write, PLCPOUType=VariableWrite, PLCOperandExpression=xxx_PLC_VAR_duty_cycle}
      mask prompts: PLCOperandTag "Data Tag:"
      mask init:
      | block = gcb;
      | maskObj = Simulink.Mask.get(block);
      | maskObj.Display = sprintf('disp(''%s'');', PLCOperandTag);
      | slplc.callbacks.initInstruction(block);
      | maskObj = Simulink.Mask.get(gcb);
      | maskObj.BlockDVGIcon = 'slplclib.VariableWrite';
      mask Help: web([docroot '/plccoder/ref/variablewrite' '.html']);
      mask DialogControl PLCBlockTitle Prompt: Data Tag Write
      mask Display: disp('duty_cycle');
  Variable Write1 : Subsystem  Priority=51  TreatAsAtomicUnit=on  mask{PLCOperandTag=OrangeLed, PLCBlockType=VariableWrite, PLCOperandAccess=Write, PLCPOUType=VariableWrite, PLCOperandExpression=xxx_PLC_VAR_OrangeLed}
      mask prompts: PLCOperandTag "Data Tag:"
      mask init: same as Lab2_simulink_plc/PLC Controller Suite/__Logic/Fukt logic/Program/__Logic/Variable Write
      mask Help: web([docroot '/plccoder/ref/variablewrite' '.html']);
      mask DialogControl PLCBlockTitle Prompt: Data Tag Write
      mask Display: disp('OrangeLed');
  Variable Write2 : Subsystem  Priority=35  TreatAsAtomicUnit=on  mask{PLCOperandTag=OrangeLed, PLCBlockType=VariableWrite, PLCOperandAccess=Write, PLCPOUType=VariableWrite, PLCOperandExpression=xxx_PLC_VAR_OrangeLed}
      mask prompts: PLCOperandTag "Data Tag:"
      mask init: same as Lab2_simulink_plc/PLC Controller Suite/__Logic/Fukt logic/Program/__Logic/Variable Write
      mask Help: web([docroot '/plccoder/ref/variablewrite' '.html']);
      mask DialogControl PLCBlockTitle Prompt: Data Tag Write
      mask Display: disp('OrangeLed');
  Variable Write3 : Subsystem  Priority=44  TreatAsAtomicUnit=on  mask{PLCOperandTag=OrangeLed, PLCBlockType=VariableWrite, PLCOperandAccess=Write, PLCPOUType=VariableWrite, PLCOperandExpression=xxx_PLC_VAR_OrangeLed}
      mask prompts: PLCOperandTag "Data Tag:"
      mask init: same as Lab2_simulink_plc/PLC Controller Suite/__Logic/Fukt logic/Program/__Logic/Variable Write
      mask Help: web([docroot '/plccoder/ref/variablewrite' '.html']);
      mask DialogControl PLCBlockTitle Prompt: Data Tag Write
      mask Display: disp('OrangeLed');
  XIC3 : Subsystem  Priority=7  TreatAsAtomicUnit=on  mask{PLCOperandTag=fukt_polling, PLCBlockType=XIC, PLCPOUType=Contact, PLCOperandExpression=xxx_PLC_VAR_fukt_polling}
      mask prompts: PLCOperandTag "Operand Tag:"
      mask init:
      | slplc.callbacks.initInstruction(gcb);
      settings: AttributesFormatString=%<PLCOperandTag>
      mask Help: web([docroot '/plccoder/ref/xic' '.html']);
      mask DialogControl PLCBlockTitle Prompt: Examine If Closed (XIC)
  XIC4 : Subsystem  Priority=48  TreatAsAtomicUnit=on  mask{PLCOperandTag=fukt_low, PLCBlockType=XIC, PLCPOUType=Contact, PLCOperandExpression=xxx_PLC_VAR_fukt_low}
      mask prompts: PLCOperandTag "Operand Tag:"
      mask init:
      | slplc.callbacks.initInstruction(gcb);
      settings: AttributesFormatString=%<PLCOperandTag>
      mask Help: web([docroot '/plccoder/ref/xic' '.html']);
      mask DialogControl PLCBlockTitle Prompt: Examine If Closed (XIC)
  XIC5 : Subsystem  Priority=47  TreatAsAtomicUnit=on  mask{PLCOperandTag=fukt_high, PLCBlockType=XIC, PLCPOUType=Contact, PLCOperandExpression=xxx_PLC_VAR_fukt_high}
      mask prompts: PLCOperandTag "Operand Tag:"
      mask init:
      | slplc.callbacks.initInstruction(gcb);
      settings: AttributesFormatString=%<PLCOperandTag>
      mask Help: web([docroot '/plccoder/ref/xic' '.html']);
      mask DialogControl PLCBlockTitle Prompt: Examine If Closed (XIC)
  XIC6 : Subsystem  Priority=41  TreatAsAtomicUnit=on  mask{PLCOperandTag=orange_led_timer_on.DN, PLCBlockType=XIC, PLCPOUType=Contact, PLCOperandExpression=xxx_PLC_VAR_orange_led_timer_on.DN}
      mask prompts: PLCOperandTag "Operand Tag:"
      mask init:
      | slplc.callbacks.initInstruction(gcb);
      settings: AttributesFormatString=%<PLCOperandTag>
      mask Help: web([docroot '/plccoder/ref/xic' '.html']);
      mask DialogControl PLCBlockTitle Prompt: Examine If Closed (XIC)
  XIO : Subsystem  Priority=29  TreatAsAtomicUnit=on  mask{PLCOperandExpression=xxx_PLC_VAR_fukt_high, PLCOperandTag=fukt_high, PLCBlockType=XIO, PLCPOUType=Contact}
      mask prompts: PLCOperandTag "Operand Tag:"
      mask init:
      | slplc.callbacks.initInstruction(gcb);
      settings: AttributesFormatString=%<PLCOperandTag>
      mask Help: web([docroot '/plccoder/ref/xio' '.html']);
      mask DialogControl PLCBlockTitle Prompt: Examine If Open (XIO)
  XIO1 : Subsystem  Priority=30  TreatAsAtomicUnit=on  mask{PLCOperandExpression=xxx_PLC_VAR_fukt_low, PLCOperandTag=fukt_low, PLCBlockType=XIO, PLCPOUType=Contact}
      mask prompts: PLCOperandTag "Operand Tag:"
      mask init:
      | slplc.callbacks.initInstruction(gcb);
      settings: AttributesFormatString=%<PLCOperandTag>
      mask Help: web([docroot '/plccoder/ref/xio' '.html']);
      mask DialogControl PLCBlockTitle Prompt: Examine If Open (XIO)
  XIO2 : Subsystem  Priority=38  TreatAsAtomicUnit=on  mask{PLCOperandExpression=xxx_PLC_VAR_fukt_high, PLCOperandTag=fukt_high, PLCBlockType=XIO, PLCPOUType=Contact}
      mask prompts: PLCOperandTag "Operand Tag:"
      mask init:
      | slplc.callbacks.initInstruction(gcb);
      settings: AttributesFormatString=%<PLCOperandTag>
      mask Help: web([docroot '/plccoder/ref/xio' '.html']);
      mask DialogControl PLCBlockTitle Prompt: Examine If Open (XIO)
  XIO3 : Subsystem  Priority=39  TreatAsAtomicUnit=on  mask{PLCOperandExpression=xxx_PLC_VAR_fukt_low, PLCOperandTag=fukt_low, PLCBlockType=XIO, PLCPOUType=Contact}
      mask prompts: PLCOperandTag "Operand Tag:"
      mask init:
      | slplc.callbacks.initInstruction(gcb);
      settings: AttributesFormatString=%<PLCOperandTag>
      mask Help: web([docroot '/plccoder/ref/xio' '.html']);
      mask DialogControl PLCBlockTitle Prompt: Examine If Open (XIO)
  XIO4 : Subsystem  Priority=32  TreatAsAtomicUnit=on  mask{PLCOperandExpression=xxx_PLC_VAR_orange_led_timer_off.DN, PLCOperandTag=orange_led_timer_off.DN, PLCBlockType=XIO, PLCPOUType=Contact}
      mask prompts: PLCOperandTag "Operand Tag:"
      mask init:
      | slplc.callbacks.initInstruction(gcb);
      settings: AttributesFormatString=%<PLCOperandTag>
      mask Help: web([docroot '/plccoder/ref/xio' '.html']);
      mask DialogControl PLCBlockTitle Prompt: Examine If Open (XIO)
  wires:
  XIC3/1(RungOut) -> LEQ/1(RungIn)
  Variable Read/1(VarOut) -> LEQ/2(srcA)
  Variable Read1/1(VarOut) -> LEQ/3(srcB)
  OTU1/1(RungOut) -> Rung Terminal2/1(RungIn)
  XIC4/1(RungOut) -> MOV/1(RungIn)
  OTL1/1(RungOut) -> OTU1/1(RungIn)
  Variable Read2/1(VarOut) -> GEQ/2(srcA)
  Variable Read3/1(VarOut) -> GEQ/3(srcB)
  LEQ/1(RungOut) -> OTL1/1(RungIn)
  Power Rail Start/1(PowerOut) -> DIV/1(RungIn), XIC3/1(RungIn), XIC5/1(RungIn), Power Rail Terminal/1(PowerIn), XIO3/1(RungIn), XIO2/1(RungIn), XIO1/1(RungIn), XIO/1(RungIn), LEQ1/1(RungIn), GEQ/1(RungIn), GEQ1/1(RungIn)
  GEQ/1(RungOut) -> OTL2/1(RungIn)
  OTL2/1(RungOut) -> Rung Terminal3/1(RungIn)
  XIC5/1(RungOut) -> XIC4/1(RungIn)
  Variable Read4/1(VarOut) -> DIV/2(srcA)
  Variable Read5/1(VarOut) -> DIV/3(srcB)
  DIV/2(dest) -> Variable Write/1(VarIn)
  DIV/1(RungOut) -> Rung Terminal4/1(RungIn)
  Variable Read6/1(VarOut) -> MOV/2(src)
  MOV/2(dest) -> Variable Write1/1(VarIn)
  XIO/1(RungOut) -> Junction/1(RungIn1)
  XIO1/1(RungOut) -> Junction/2(RungIn2)
  MOV1/2(dest) -> Variable Write2/1(VarIn)
  Variable Read7/1(VarOut) -> MOV1/2(src)
  MOV1/1(RungOut) -> TON1/1(RungIn)
  Junction/1(RungOut) -> XIO4/1(RungIn)
  XIO3/1(RungOut) -> Junction1/2(RungIn2)
  XIO2/1(RungOut) -> Junction1/1(RungIn1)
  Junction1/1(RungOut) -> XIC6/1(RungIn)
  Variable Read8/1(VarOut) -> MOV2/2(src)
  MOV2/2(dest) -> Variable Write3/1(VarIn)
  XIC6/1(RungOut) -> MOV2/1(RungIn)
  MOV2/1(RungOut) -> TON2/1(RungIn)
  XIO4/1(RungOut) -> MOV1/1(RungIn)
  TON1/1(EN) -> Rung Terminal5/1(RungIn)
  TON2/1(EN) -> Rung Terminal6/1(RungIn)
  MOV/1(RungOut) -> Rung Terminal1/1(RungIn)
  Variable Read9/1(VarOut) -> GEQ1/2(srcA)
  Variable Read10/1(VarOut) -> GEQ1/3(srcB)
  GEQ1/1(RungOut) -> OTU2/1(RungIn)
  OTU2/1(RungOut) -> Rung Terminal7/1(RungIn)
  LEQ1/1(RungOut) -> OTU3/1(RungIn)
  OTU3/1(RungOut) -> Rung Terminal8/1(RungIn)
  Variable Read12/1(VarOut) -> LEQ1/3(srcB)
  Variable Read11/1(VarOut) -> LEQ1/2(srcA)
